simulink model "MCVT_Integration"  (R2020a)
settings: Solver=ode8  StartTime=0.0  StopTime=T_end  FixedStep=dt  RelTol=1e-3  EnableMultiTasking=on
values from neighbouring files (symbols this model uses):
  assumed_power_voltage = 1.25e2    (= 125)    [MCVT_Input_File.m]    (also in MCVT_Run_QuickCheck.m)
  battery_power_toggle = 1    [MCVT_Input_File.m]    (also in MCVT_Run_QuickCheck.m)
  battery_trickle_charge_param = 0.1    [MCVT_Input_File.m]    (also in MCVT_Run_QuickCheck.m)
  battery_trickle_leakage_param = 0.01    [MCVT_Input_File.m]    (also in MCVT_Run_QuickCheck.m)
  damage_case_id = 3    [MCVT_Input_File.m]    (also in MCVT_Run_QuickCheck.m = 1)
  dt = 1/fs    (= 0.001)    [MCVT_Run_QuickCheck.m]    (also in MCVT_Sim_Set.m)
  dt_2 = 1/fs_2    (= 0.001)    [MCVT_Run_QuickCheck.m]    (also in MCVT_Sim_Set.m)
  dt_3 = 1/fs_3    (= 0.001)    [MCVT_Run_QuickCheck.m]    (also in MCVT_Sim_Set.m)
  dt_5 = 1/fs_5    (= 0.005)    [MCVT_Run_QuickCheck.m]    (also in MCVT_Sim_Set.m)
  dt_8 = 1/fs_8    (= 0.05)    [MCVT_Run_QuickCheck.m]    (also in MCVT_Sim_Set.m)
  dt_9 = 1/fs_9    (= 0.001)    [MCVT_Run_QuickCheck.m]    (also in MCVT_Sim_Set.m)
  dust_met_duration = 3    [MCVT_Input_File.m]    (also in MCVT_Run_QuickCheck.m = 2)
  ECLSS_indoor_set_temperature = 298.15    [MCVT_Input_File.m]    (also in MCVT_Run_QuickCheck.m)
  energy_store_max_o = 150    [MCVT_Input_File.m]    (also in MCVT_Run_QuickCheck.m)
  energy_store_o = 120    [MCVT_Input_File.m]    (also in MCVT_Run_QuickCheck.m)
  fs = 1000    [MCVT_Run_QuickCheck.m]    (also in MCVT_Sim_Set.m)
  gain_charge_param = 1    [MCVT_Input_File.m]    (also in MCVT_Run_QuickCheck.m)
  ground_temperature = 373    [MCVT_Habitat_Design_Input_File.m]    (also in MCVT_Run_QuickCheck.m)
  I_max_charge = 800    [MCVT_Input_File.m]    (also in MCVT_Run_QuickCheck.m)
  I_max_discharge = 800    [MCVT_Input_File.m]    (also in MCVT_Run_QuickCheck.m)
  initial_pressure = 1e5    (= 100000)    [MCVT_Input_File.m]    (also in MCVT_Run_QuickCheck.m)
  initial_temperature = 295    [MCVT_Input_File.m]    (also in MCVT_Run_QuickCheck.m)
  interior_leak_rate = 0.00415    [MCVT_Input_File.m]    (also in MCVT_Run_QuickCheck.m)
  launch_dust_accum_start_time = 10    [MCVT_Input_File.m]    (also in MCVT_Run_QuickCheck.m = 2)
  launch_dust_accum_stop_time = 12    [MCVT_Input_File.m]    (also in MCVT_Run_QuickCheck.m = 3)
  meteorite_impact_time = 2    [MCVT_Input_File.m]    (also in MCVT_Run_QuickCheck.m = 1)
  nuclear_power_toggle = 1    [MCVT_Input_File.m]    (also in MCVT_Run_QuickCheck.m)
  print_interval = 30    [MCVT_Input_File.m]
  SOC_lower_thres = 0.5    [MCVT_Input_File.m]    (also in MCVT_Run_QuickCheck.m)
  SOC_upper_thres = 0.9    [MCVT_Input_File.m]    (also in MCVT_Run_QuickCheck.m)
  solar_power_toggle = 1    [MCVT_Input_File.m]    (also in MCVT_Run_QuickCheck.m)
  space_temperature = 4    [MCVT_Habitat_Design_Input_File.m]    (also in MCVT_Run_QuickCheck.m)
  Start_angle = 90    [MCVT_Input_File.m]    (also in MCVT_Run_QuickCheck.m)
undefined symbols (not defined in the file or in its folder): A_flow_restrictor, ACTIVITY_LOOKUP_TABLE, air, Aref, Bmap, Bmap2, Bmap3, comms, DiscIntNeverNeededParam, DmgSPL, dust_parameters, ECLSS_dust_damage_repair_time, ECLSS_heater_capacity, ECLSS_IPS_restrictor, ECLSS_IPS_setpointPressure, ECLSS_paint_damage_repair_time, ECLSS_radiatorLoop_discreteNumber, ECLSS_Vst, emptyActivity, expected_heat_add, FI, habitat, HIEM2SMM, HIEM2STM, HIEMDamageElements, impact_numbering, initial_SPLT_damage_index, input_air, input_Cond, input_CP, input_discretization, input_EV, input_Evap, input_radiatorLoop, K_ECLSS_dust_single, K_ECLSS_paint_single, K_Structure_damage_single, Kref, kW_to_W, meteoroidDustDep, mFRF, ndelays, nominalDustDep, noverlap, nuclear_toggle_gain, output_limits, panel_paint0, Ps0, reference_temperature, RepairTime_ECLSS_factor, Sensor_Location_SMM, SMM_T0, SMM_T1, SMM_T2, SMM_T3, SMM_T4, SMM_T4_0, SMM_T4_1, SMM_T4_2, solar_FDD_K ... (+73)
libraries: neural x350, simulink x39, xpcutilitieslib x7, xpcrtudplib x7, dspstat3 x1
(part 25 of 43)

== system MCVT_Integration/Sys2 - Structural System/Sys2_Structural_System/Fault Detection and Diagnosis (FDD) with Binary Classifer/Pattern Recognition Neural Network/Segment 2 (2)/Layer 2/Delays 1 ==
  "p{2}" : Inport  PortDimensions=20
      mask Display: e = 0.06;c1 = [120 230 180]/255;c2 = ([120 230 180]+0)/(256+128);c3 = ([120 230 180]+128)/(256+128);patch([0 1 1 0],[0 0 1 1],c1);patch([0 1 1 1-e 1-e 0+e],[0 0 1 1-e 0+e 0+e],c2);patch([0 0 1 1-e 0+e 0+e],[0 1 1 1-e 1-e 0+e],c3);plot([0 1 1 0 0],[0 0 1 1 0]);
  mux : Mux  Inputs=1
      mask Display: e = 0.06;c1 = [120 150 230]/255;c2 = ([120 150 230]+0)/383;c3 = ([120 150 230]+128)/383;patch([0 1 1 0],[0 0 1 1],c1);patch([0 1 1 1-e 1-e 0+e],[0 0 1 1-e 0+e 0+e],c2);patch([0 0 1 1-e 0+e 0+e],[0 1 1 1-e 1-e 0+e],c3);plot([0 1 1 0 0],[0 0 1 1 0]);disp('Mux')
  "pd{2,1}" : Outport
      mask Display: e = 0.06;c1 = [120 230 180]/255;c2 = ([120 230 180]+0)/(256+128);c3 = ([120 230 180]+128)/(256+128);patch([0 1 1 0],[0 0 1 1],c1);patch([0 1 1 1-e 1-e 0+e],[0 0 1 1-e 0+e 0+e],c2);patch([0 0 1 1-e 0+e 0+e],[0 1 1 1-e 1-e 0+e],c3);plot([0 1 1 0 0],[0 0 1 1 0]);
  wires:
  "p{2}"/1 -> mux/1
  mux/1 -> "pd{2,1}"/1

== system MCVT_Integration/Sys2 - Structural System/Sys2_Structural_System/Fault Detection and Diagnosis (FDD) with Binary Classifer/Pattern Recognition Neural Network/Segment 2 (2)/Layer 2/LW{2,1} ==
  "ad{2,1}" : Inport  PortDimensions=20
      mask Display: e = 0.06;c1 = [120 230 180]/255;c2 = ([120 230 180]+0)/(256+128);c3 = ([120 230 180]+128)/(256+128);patch([0 1 1 0],[0 0 1 1],c1);patch([0 1 1 1-e 1-e 0+e],[0 0 1 1-e 0+e 0+e],c2);patch([0 0 1 1-e 0+e 0+e],[0 1 1 1-e 1-e 0+e],c3);plot([0 1 1 0 0],[0 0 1 1 0]);
  "IW{2,1}(1,:)'" : Constant  Value=[-0.0131519501662464843860789187601767480373382568359375;-0.2283490556409899785439421293631312437355518341064453125;-0.289726021936160205161314706856501288712024688720703125;-0.610136834219936030621056488598696887493133544921875;-0.2034645657655405892594302486031665466725826263427734375;-0.1781002138410512636301774591629509814083576202392578125;-0.29252056118321412281346738382126204669475555419921875;0.62775860490154566928566737260553054511547088623046875;0.436139315774980607098854079595184884965419769287109375;-0.09668622512830470083766698508043191395699977874755859375;-0.117092883490729604556435106132994405925273895263671875;-0.50352244145249358542315576414694078266620635986328125;0.447763606238912259538409443848649971187114715576171875;0.16290226867061274873549336916767060756683349609375;-0.07475694900080988303248119564159424044191837310791015625;0.10059141232051337089270504066007561050355434417724609375;0.392832502066031874488061248484882526099681854248046875;-0.37563150969789649291641353556769900023937225341796875;0.6032049190998052257128847486455924808979034423828125;0.5332559068353577291787814829149283468723297119140625]
      mask Display: e = 0.06;c1 = [120 150 230]/255;c2 = ([120 150 230]+0)/383;c3 = ([120 150 230]+128)/383;patch([0 1 1 0],[0 0 1 1],c1);patch([0 1 1 1-e 1-e 0+e],[0 0 1 1-e 0+e 0+e],c2);patch([0 0 1 1-e 0+e 0+e],[0 1 1 1-e 1-e 0+e],c3);plot([0 1 1 0 0],[0 0 1 1 0]);disp('weights');
  "IW{2,1}(10,:)'" : Constant  Value=[0.197300923241983838796187455955077894032001495361328125;-0.09049196986361050798475247347596450708806514739990234375;-0.14234633867032464582536022135172970592975616455078125;-0.3499922447867491737127920714556239545345306396484375;-0.12360098816561958245952013157875626347959041595458984375;-0.53617351984011907273242059090989641845226287841796875;-0.63019586873835731211102029192261397838592529296875;-0.59953072347420388599203988633234985172748565673828125;-0.0963323722927145464911546923758578486740589141845703125;0.31476140520503637088012283129501156508922576904296875;0.1658873255039448324499318232483346946537494659423828125;-0.5239396673414631333542956781457178294658660888671875;-0.359447552274538340011389436767785809934139251708984375;-0.52141836566016053211569669656455516815185546875;0.1987488184022156989527729820110835134983062744140625;0.13545688063019600821945687130209989845752716064453125;-0.390371505202162349945638197823427617549896240234375;-0.330714938560074944717825928819365799427032470703125;0.195111837513341768168828593843500129878520965576171875;0.477428590936654184684329038645955733954906463623046875]
      mask Display: e = 0.06;c1 = [120 150 230]/255;c2 = ([120 150 230]+0)/383;c3 = ([120 150 230]+128)/383;patch([0 1 1 0],[0 0 1 1],c1);patch([0 1 1 1-e 1-e 0+e],[0 0 1 1-e 0+e 0+e],c2);patch([0 0 1 1-e 0+e 0+e],[0 1 1 1-e 1-e 0+e],c3);plot([0 1 1 0 0],[0 0 1 1 0]);disp('weights');
  "IW{2,1}(11,:)'" : Constant  Value=[-0.43816409613136875211836240850971080362796783447265625;0.050414382838295558608709967529648565687239170074462890625;0.3709541683366754938333542668260633945465087890625;0.362179073798906348002901722793467342853546142578125;-0.477214101448056371435058053975808434188365936279296875;0.09154699814056588280664072954095900058746337890625;-0.507130106053504281504729078733362257480621337890625;-0.1507137484925420156667286164520191960036754608154296875;0.275939301654270441321870066531118936836719512939453125;-0.2303026309926386738791137531734420917928218841552734375;-0.370681237269094354669363156062900088727474212646484375;0.26264946901190366901346351369284093379974365234375;-0.35779494535728095794269165708101354539394378662109375;0.276947799384692883162273346897563897073268890380859375;-0.529013627469939518022101765382103621959686279296875;0.498452822401328765611339122187928296625614166259765625;-0.52921250233643568616770380685920827090740203857421875;-0.2138755050931575862893652129059773869812488555908203125;0.1237822832115683391318583517204388044774532318115234375;-0.52223863473583331806793239593389444053173065185546875]
      mask Display: e = 0.06;c1 = [120 150 230]/255;c2 = ([120 150 230]+0)/383;c3 = ([120 150 230]+128)/383;patch([0 1 1 0],[0 0 1 1],c1);patch([0 1 1 1-e 1-e 0+e],[0 0 1 1-e 0+e 0+e],c2);patch([0 0 1 1-e 0+e 0+e],[0 1 1 1-e 1-e 0+e],c3);plot([0 1 1 0 0],[0 0 1 1 0]);disp('weights');
  "IW{2,1}(12,:)'" : Constant  Value=[-0.154179751547239674014377897037775255739688873291015625;0.24132279555188151931588436127640306949615478515625;-0.1907832451890008884642924158470123074948787689208984375;-0.1898471638859701438040161747267120517790317535400390625;0.08850062513875656122763757593929767608642578125;-0.09830670129288728020799936757612158544361591339111328125;-0.3458340449024610574468852064455859363079071044921875;0.58622057419220741092402704452979378402233123779296875;-0.096689363847558051912045584685984067618846893310546875;-0.121243727115140698114004180752090178430080413818359375;-0.34262826548283153993423866268130950629711151123046875;0.42695656487580613269727791703189723193645477294921875;0.0409180102003559864609627538811764679849147796630859375;-0.215755452435445416181636346664163284003734588623046875;-0.368713595036108288116594167149742133915424346923828125;-0.63218708471201845444653599770390428602695465087890625;0.54504381690632286439068820982356555759906768798828125;0.5238956902929248204969780999817885458469390869140625;0.58609328606454325072405708851874805986881256103515625;0.43090807344199133499529352775425650179386138916015625]
      mask Display: e = 0.06;c1 = [120 150 230]/255;c2 = ([120 150 230]+0)/383;c3 = ([120 150 230]+128)/383;patch([0 1 1 0],[0 0 1 1],c1);patch([0 1 1 1-e 1-e 0+e],[0 0 1 1-e 0+e 0+e],c2);patch([0 0 1 1-e 0+e 0+e],[0 1 1 1-e 1-e 0+e],c3);plot([0 1 1 0 0],[0 0 1 1 0]);disp('weights');
  "IW{2,1}(13,:)'" : Constant  Value=[-0.30546889022732603979903842628118582069873809814453125;0.51596770552269377585474785519181750714778900146484375;-0.34669813473577004714343274827115237712860107421875;0.12420386955850241939014466652224655263125896453857421875;0.63521116212299399972351920951041392982006072998046875;0.141702815706782114801853822427801787853240966796875;-0.04114993903501988625759366868805955164134502410888671875;-0.01341912357002485005341707591242084163241088390350341796875;-0.2256961036674860476836101952358148992061614990234375;0.07905006322392633955065122108862851746380329132080078125;0.789135755776883218715056500514037907123565673828125;0.00699798297809421480930591741298485430888831615447998046875;0.7010967977420377028607845204533077776432037353515625;0.1653077210484481252006361273743095807731151580810546875;-0.138169087998334438882608310450450517237186431884765625;0.32630544085148105448723754307138733565807342529296875;-0.041991616347027027611460425760014913976192474365234375;0.635256180584609086992031734553165733814239501953125;-0.1329167055636059913137358989843050949275493621826171875;0.031908811545528194264509380673189298249781131744384765625]
      mask Display: e = 0.06;c1 = [120 150 230]/255;c2 = ([120 150 230]+0)/383;c3 = ([120 150 230]+128)/383;patch([0 1 1 0],[0 0 1 1],c1);patch([0 1 1 1-e 1-e 0+e],[0 0 1 1-e 0+e 0+e],c2);patch([0 0 1 1-e 0+e 0+e],[0 1 1 1-e 1-e 0+e],c3);plot([0 1 1 0 0],[0 0 1 1 0]);disp('weights');
  "IW{2,1}(14,:)'" : Constant  Value=[0.67168627283706328068291213639895431697368621826171875;0.1960581153137800625163578160936594940721988677978515625;0.1827421028051608165032604347288724966347217559814453125;-0.197999380927649848427307688325527124106884002685546875;-0.5284048661146687475564931446569971740245819091796875;-0.52095844209163544302754189629922620952129364013671875;0.2375769278955271179842867468323674984276294708251953125;0.413627245104900620464860594438505358994007110595703125;0.1650150353793438939842275203773169778287410736083984375;0.00104023284521608254567237583643191101145930588245391845703125;0.4547232127345341989865801224368624389171600341796875;0.1755674189985755584419990782407694496214389801025390625;0.396113807751520552447033196585834957659244537353515625;-0.37449472969020780599436193369911052286624908447265625;0.548398537903627580902821136987768113613128662109375;0.3822967344072323303549865158856846392154693603515625;0.258528703254273295453202763383160345256328582763671875;0.14519592981383999674704909921274520456790924072265625;0.333280739538195813764787089894525706768035888671875;-0.418880074668204371857171963711152784526348114013671875]
      mask Display: e = 0.06;c1 = [120 150 230]/255;c2 = ([120 150 230]+0)/383;c3 = ([120 150 230]+128)/383;patch([0 1 1 0],[0 0 1 1],c1);patch([0 1 1 1-e 1-e 0+e],[0 0 1 1-e 0+e 0+e],c2);patch([0 0 1 1-e 0+e 0+e],[0 1 1 1-e 1-e 0+e],c3);plot([0 1 1 0 0],[0 0 1 1 0]);disp('weights');
  "IW{2,1}(15,:)'" : Constant  Value=[0.204965259719101233759630531494622118771076202392578125;-0.457528895464862361830427062159287743270397186279296875;0.396842705297933140418109587699291296303272247314453125;-0.209056454484637221913345683788065798580646514892578125;0.39452448007547558095353679163963533937931060791015625;-0.00080403082643378041664827815537819333258084952831268310546875;-0.30421576480985346169205740807228721678256988525390625;-0.40593922296600981614034253652789629995822906494140625;0.167199455682886022334088238494587130844593048095703125;0.37516990390103444052982695211539976298809051513671875;0.04725408586966405033447102823629393242299556732177734375;-0.2294834142945360311305336153964162804186344146728515625;0.055303747495165346548251505964799434877932071685791015625;-0.58871003988118875138724206408369354903697967529296875;0.63547097898882476396664742424036376178264617919921875;-0.5822402191211561994776957362773828208446502685546875;0.386993895216280192617119837450445629656314849853515625;0.322000985675978956823684029586729593575000762939453125;-0.44334798489222648765206713505904190242290496826171875;-0.289612766679017308302235278461012057960033416748046875]
      mask Display: e = 0.06;c1 = [120 150 230]/255;c2 = ([120 150 230]+0)/383;c3 = ([120 150 230]+128)/383;patch([0 1 1 0],[0 0 1 1],c1);patch([0 1 1 1-e 1-e 0+e],[0 0 1 1-e 0+e 0+e],c2);patch([0 0 1 1-e 0+e 0+e],[0 1 1 1-e 1-e 0+e],c3);plot([0 1 1 0 0],[0 0 1 1 0]);disp('weights');
  "IW{2,1}(16,:)'" : Constant  Value=[0.5086877522888617608742833908763714134693145751953125;0.155454499671700385743378092229249887168407440185546875;0.298448023683270335926209781973739154636859893798828125;0.21427800561056697947748261867673136293888092041015625;0.255418943293809486139167574947350658476352691650390625;0.2022610655742179408544956231708056293427944183349609375;0.566119820861264333444751173374243080615997314453125;0.52729264426775224183785439890925772488117218017578125;0.013144346486769355186741137231365428306162357330322265625;-0.40351641591171005796212511995690874755382537841796875;0.329441369614369872209636014304123818874359130859375;0.292127223318678741481591032425058074295520782470703125;0.409605478422182367470583130852901376783847808837890625;-0.480182871235225350314834713572054170072078704833984375;-0.5012470349158772986442045294097624719142913818359375;0.1676099786342380715264965829192078672349452972412109375;0.1959335407295708930686117810182622633874416351318359375;-0.447260994875497031042499429531744681298732757568359375;0.51517899022007984655147083685733377933502197265625;-0.340207096308376477633572676495532505214214324951171875]
      mask Display: e = 0.06;c1 = [120 150 230]/255;c2 = ([120 150 230]+0)/383;c3 = ([120 150 230]+128)/383;patch([0 1 1 0],[0 0 1 1],c1);patch([0 1 1 1-e 1-e 0+e],[0 0 1 1-e 0+e 0+e],c2);patch([0 0 1 1-e 0+e 0+e],[0 1 1 1-e 1-e 0+e],c3);plot([0 1 1 0 0],[0 0 1 1 0]);disp('weights');
  "IW{2,1}(17,:)'" : Constant  Value=[0.2441649229961604106176054074239800684154033660888671875;-0.44964204744072777675256702423212118446826934814453125;-0.475088415645347483806659738547750748693943023681640625;-0.195526594771391282190364790949388407170772552490234375;0.29813918813556294917788136444869451224803924560546875;-0.4409374283631042334974381446954794228076934814453125;0.5065848325108703864572134989430196583271026611328125;0.35097759710480713746250103213242255151271820068359375;-0.2567248005003490707309765639365650713443756103515625;0.38262506906693538599739667915855534374713897705078125;0.1345017254853722865615139880901551805436611175537109375;0.51895290240209501764212518537533469498157501220703125;0.4519398832964345302798392367549240589141845703125;0.133895624946028413138066071041976101696491241455078125;-0.2060589941360758403643416158956824801862239837646484375;-0.397105489244936660231388714237255044281482696533203125;-0.4186089314300984742800437743426300585269927978515625;0.426739301003949578205975967648555524647235870361328125;0.446382722894400008062376628004130907356739044189453125;-0.213927716619095209527046108632930554449558258056640625]
      mask Display: e = 0.06;c1 = [120 150 230]/255;c2 = ([120 150 230]+0)/383;c3 = ([120 150 230]+128)/383;patch([0 1 1 0],[0 0 1 1],c1);patch([0 1 1 1-e 1-e 0+e],[0 0 1 1-e 0+e 0+e],c2);patch([0 0 1 1-e 0+e 0+e],[0 1 1 1-e 1-e 0+e],c3);plot([0 1 1 0 0],[0 0 1 1 0]);disp('weights');
  "IW{2,1}(18,:)'" : Constant  Value=[-0.0272818523825677609895290487429519998840987682342529296875;-0.1992454448341149098045121945688151754438877105712890625;0.00399563298715272137429099785776998032815754413604736328125;-0.49122836298554217737688531997264362871646881103515625;0.26900137384623068470546058961190283298492431640625;0.419204027612011753678444847537321038544178009033203125;-0.56941485889710496959281726958579383790493011474609375;0.59417278501397008394491194849251769483089447021484375;-0.01317092597891093841422360810611280612647533416748046875;0.498478065508463463739730059387511573731899261474609375;-0.1365275002181844110626940391739481128752231597900390625;0.349094615244758987859796661723521538078784942626953125;0.333932621740755941619482882742886431515216827392578125;0.236908066657262217358237421649391762912273406982421875;-0.272717938937628023854387038227287121117115020751953125;-0.267866598781879738933042744974954985082149505615234375;0.47520848350665001458281722079846076667308807373046875;-0.1413815765253161049752606004403787665069103240966796875;-0.28032347811350588617784751477302052080631256103515625;-0.6554303965414323140947772117215208709239959716796875]
      mask Display: e = 0.06;c1 = [120 150 230]/255;c2 = ([120 150 230]+0)/383;c3 = ([120 150 230]+128)/383;patch([0 1 1 0],[0 0 1 1],c1);patch([0 1 1 1-e 1-e 0+e],[0 0 1 1-e 0+e 0+e],c2);patch([0 0 1 1-e 0+e 0+e],[0 1 1 1-e 1-e 0+e],c3);plot([0 1 1 0 0],[0 0 1 1 0]);disp('weights');
  "IW{2,1}(19,:)'" : Constant  Value=[-0.412984680238038748267825894799898378551006317138671875;-0.01197734433299585919685537049872436909936368465423583984375;-0.41675158842994586283481339705758728086948394775390625;0.572292832534660345089605471002869307994842529296875;-0.59742353013398930006161435812828131020069122314453125;0.320138578299695664153290408648899756371974945068359375;-0.23180110089244287951970591166173107922077178955078125;-0.0032164649152252818876951412363496274338103830814361572265625;-0.04897654664051438733185506180234369821846485137939453125;0.27541941282966686532773792350781150162220001220703125;-0.185825929747873541675318165289354510605335235595703125;0.096966390649146472213715242105536162853240966796875;0.54300070707530168068188913821359165012836456298828125;0.6121233500942977467929040358285419642925262451171875;0.53229438575759047136415347267757169902324676513671875;0.1371221791612284024441947849481948651373386383056640625;0.337732805587329820706798955143312923610210418701171875;-0.471249189997828554776759801825392059981822967529296875;0.1414943335377516209216963716244208626449108123779296875;-0.260606610726190235372001779978745616972446441650390625]
      mask Display: e = 0.06;c1 = [120 150 230]/255;c2 = ([120 150 230]+0)/383;c3 = ([120 150 230]+128)/383;patch([0 1 1 0],[0 0 1 1],c1);patch([0 1 1 1-e 1-e 0+e],[0 0 1 1-e 0+e 0+e],c2);patch([0 0 1 1-e 0+e 0+e],[0 1 1 1-e 1-e 0+e],c3);plot([0 1 1 0 0],[0 0 1 1 0]);disp('weights');
  "IW{2,1}(2,:)'" : Constant  Value=[0.333457618335939509623955245842807926237583160400390625;0.31260328922987612454420514040975831449031829833984375;0.079716604354632514262135600802139379084110260009765625;0.26287435946747350623553529658238403499126434326171875;0.307987751629796802088634422034374438226222991943359375;-0.434530480747118363904490934146451763808727264404296875;-0.1955650245888486959966456879556062631309032440185546875;0.0316630319989695541149643531753099523484706878662109375;0.149008003242111553010573743449640460312366485595703125;-0.1762212999549400971321944098235690034925937652587890625;-0.549049382692389453808345933794043958187103271484375;-0.007927998603949625022746516833649366162717342376708984375;0.04347451355695024222125510959813254885375499725341796875;0.5942988052607638405078205323661677539348602294921875;0.4983561964854132941837860926170833408832550048828125;-0.1307877313259060148009638169241952709853649139404296875;0.58851957543416444362804895717999897897243499755859375;0.58966588037519584997170341011951677501201629638671875;0.27866051602814623233683732905774377286434173583984375;0.5400702859147890055879770443425513803958892822265625]
      mask Display: e = 0.06;c1 = [120 150 230]/255;c2 = ([120 150 230]+0)/383;c3 = ([120 150 230]+128)/383;patch([0 1 1 0],[0 0 1 1],c1);patch([0 1 1 1-e 1-e 0+e],[0 0 1 1-e 0+e 0+e],c2);patch([0 0 1 1-e 0+e 0+e],[0 1 1 1-e 1-e 0+e],c3);plot([0 1 1 0 0],[0 0 1 1 0]);disp('weights');
  "IW{2,1}(20,:)'" : Constant  Value=[0.37774749543291308295778208048432134091854095458984375;0.450209832735798787251013663990306667983531951904296875;-0.01815944383427547936804558048606850206851959228515625;-0.322358661882434838208411065352265723049640655517578125;0.3101929148373734346222363456035964190959930419921875;0.28410063058146717285268323394120670855045318603515625;-0.261028404079189424447093870185199193656444549560546875;0.0238287955920568998713360286956230993382632732391357421875;0.1632484893172323758836483875711564905941486358642578125;-0.59584478130304330267819068467360921204090118408203125;-0.51739031850990857552829993437626399099826812744140625;0.45758039328849431370827005594037473201751708984375;0.497997300177656310182783272466622292995452880859375;0.34127307260028449942268480299389921128749847412109375;-0.39211144835421907206551850322284735739231109619140625;0.187366874416645179390883413361734710633754730224609375;-0.527069786600139256194097470142878592014312744140625;0.066239801981011814380195801277295686304569244384765625;0.49808832985547246696711454205797053873538970947265625;0.171781567452022498887487245156080462038516998291015625]
      mask Display: e = 0.06;c1 = [120 150 230]/255;c2 = ([120 150 230]+0)/383;c3 = ([120 150 230]+128)/383;patch([0 1 1 0],[0 0 1 1],c1);patch([0 1 1 1-e 1-e 0+e],[0 0 1 1-e 0+e 0+e],c2);patch([0 0 1 1-e 0+e 0+e],[0 1 1 1-e 1-e 0+e],c3);plot([0 1 1 0 0],[0 0 1 1 0]);disp('weights');
  "IW{2,1}(3,:)'" : Constant  Value=[0.35439732233827825336192063332418911159038543701171875;-0.313091430040572660242759184257010929286479949951171875;0.12452339499336524675232595882334862835705280303955078125;0.40565264515026833169741848905687220394611358642578125;0.57658539475180392575026644408353604376316070556640625;-0.08658277145044911138338505907086073420941829681396484375;-0.596227988176643908246887804125435650348663330078125;0.268082521162564424432872556280926801264286041259765625;-0.07489967915217420058038300112457363866269588470458984375;0.2481297207565101581305810896083130501210689544677734375;0.2074183065167887474178343154562753625214099884033203125;0.294677077928551744978591386825428344309329986572265625;-0.56237737470870763356600718907429836690425872802734375;-0.183562744032721936182639410617412067949771881103515625;0.481266272701765307484578215735382400453090667724609375;-0.33702111782774923920413812084007076919078826904296875;-0.487107004605000282193572047617635689675807952880859375;0.4731483956925763845191568179870955646038055419921875;-0.036816182760127623418977549363262369297444820404052734375;-0.34504886566515347023909043855383060872554779052734375]
      mask Display: e = 0.06;c1 = [120 150 230]/255;c2 = ([120 150 230]+0)/383;c3 = ([120 150 230]+128)/383;patch([0 1 1 0],[0 0 1 1],c1);patch([0 1 1 1-e 1-e 0+e],[0 0 1 1-e 0+e 0+e],c2);patch([0 0 1 1-e 0+e 0+e],[0 1 1 1-e 1-e 0+e],c3);plot([0 1 1 0 0],[0 0 1 1 0]);disp('weights');
  "IW{2,1}(4,:)'" : Constant  Value=[-0.386695633926689819048760909936390817165374755859375;0.255337156497296657864382041225326247513294219970703125;0.311559038575999147635542385614826343953609466552734375;-0.171833764302430302972624076573993079364299774169921875;0.42815980888437732421181181052816100418567657470703125;0.1841038622537757574182393227602005936205387115478515625;-0.514405240272733710327202061307616531848907470703125;-0.6114171015452611879226196833769790828227996826171875;0.2549273615071883103411209958721883594989776611328125;-0.358671088136504001564475174745894037187099456787109375;0.2271734734148021794819527485742582939565181732177734375;-0.50116453450551678994173698811209760606288909912109375;0.0279531759745337386735553053540570545010268688201904296875;0.2470897484313333281047420086906640790402889251708984375;-0.161814456109800886007832332325051538646221160888671875;-0.53806900757107822830249688195181079208850860595703125;-0.427958005212859593502372490547713823616504669189453125;0.388009745019373741836687941031414084136486053466796875;0.477700509700453801453789992592646740376949310302734375;-0.102658369668155058374026111778221093118190765380859375]
      mask Display: e = 0.06;c1 = [120 150 230]/255;c2 = ([120 150 230]+0)/383;c3 = ([120 150 230]+128)/383;patch([0 1 1 0],[0 0 1 1],c1);patch([0 1 1 1-e 1-e 0+e],[0 0 1 1-e 0+e 0+e],c2);patch([0 0 1 1-e 0+e 0+e],[0 1 1 1-e 1-e 0+e],c3);plot([0 1 1 0 0],[0 0 1 1 0]);disp('weights');
  "IW{2,1}(5,:)'" : Constant  Value=[-0.2183548213776102253635968963862978853285312652587890625;-0.06686080163682102395217299317664583213627338409423828125;-0.3059856159244294104837535996921360492706298828125;0.50628347562946640625369809640687890350818634033203125;-0.059884992527261891870349330702083534561097621917724609375;-0.42947409214421894407820445849210955202579498291015625;0.00577882712320255086113629516830769716762006282806396484375;0.57894313286325782552665941693703643977642059326171875;-0.228878604361737847394664413513964973390102386474609375;0.38508070925969029030255796897108666598796844482421875;-0.56889230433675719300623541130335070192813873291015625;-0.0214034368517706762025110123204285628162324428558349609375;0.2011680864736654361646372990435338579118251800537109375;0.07659518359337459669777814497138024307787418365478515625;0.58426152416850152260252571068122051656246185302734375;0.261441142296763684438332120407721959054470062255859375;-0.473890859988484181020140795226325280964374542236328125;-0.038417399100486700203038736844973755069077014923095703125;0.59236811667310129703167831394239328801631927490234375;-0.397314077343586535828734440656262449920177459716796875]
      mask Display: e = 0.06;c1 = [120 150 230]/255;c2 = ([120 150 230]+0)/383;c3 = ([120 150 230]+128)/383;patch([0 1 1 0],[0 0 1 1],c1);patch([0 1 1 1-e 1-e 0+e],[0 0 1 1-e 0+e 0+e],c2);patch([0 0 1 1-e 0+e 0+e],[0 1 1 1-e 1-e 0+e],c3);plot([0 1 1 0 0],[0 0 1 1 0]);disp('weights');
  "IW{2,1}(6,:)'" : Constant  Value=[-0.52038816182933345100281030681799165904521942138671875;-0.47827678462462552833045492661767639219760894775390625;-0.012618800528797657900526019147946499288082122802734375;-0.51684940007109048121236583028803579509258270263671875;0.5007574950134270341806086435099132359027862548828125;0.44522443493419039395320169205660931766033172607421875;-0.03644083145788785949026333810252253897488117218017578125;0.51043943242322165065871786282514221966266632080078125;-0.378811203678414809559171771979890763759613037109375;0.5236463638309718504615375422872602939605712890625;0.0476719440912919123487512251813313923776149749755859375;-0.11901798838266962332621545783695182763040065765380859375;-0.368746150437196662519312440053909085690975189208984375;-0.149549170352473448364349906114512123167514801025390625;-0.08908356480650823916445091299465275369584560394287109375;-0.337019281818837501685237612036871723830699920654296875;-0.00727271919906051500515076213559950701892375946044921875;-0.58588010866313255942117166341631673276424407958984375;-0.039562114831076723719949228552650311030447483062744140625;0.327220002377005492188999369318480603396892547607421875]
      mask Display: e = 0.06;c1 = [120 150 230]/255;c2 = ([120 150 230]+0)/383;c3 = ([120 150 230]+128)/383;patch([0 1 1 0],[0 0 1 1],c1);patch([0 1 1 1-e 1-e 0+e],[0 0 1 1-e 0+e 0+e],c2);patch([0 0 1 1-e 0+e 0+e],[0 1 1 1-e 1-e 0+e],c3);plot([0 1 1 0 0],[0 0 1 1 0]);disp('weights');
  "IW{2,1}(7,:)'" : Constant  Value=[-0.427274467634338350752187807302107103168964385986328125;0.349354643114616225663127124789752997457981109619140625;-0.45156490884748212710775305822608061134815216064453125;-0.247476066573397657233357449513277970254421234130859375;-0.333650397681086896550795017901691608130931854248046875;-0.50567896573378090518957606036565266549587249755859375;0.2867711824106915496912506569060496985912322998046875;0.084802900833068350916477129430859349668025970458984375;-0.477034292082058553052803517857682891190052032470703125;0.10820511835584674387167325448899646289646625518798828125;0.470801628314395426144756129360757768154144287109375;0.26092044910748846131554046223754994571208953857421875;7.601676874353815831220071430607276852242648601531982421875e-05;-0.1250307582448890730830726170097477734088897705078125;0.4440635884883621375962547972449101507663726806640625;-0.310202392049235997628642280687927268445491790771484375;-0.1241160455325439337936899164560600183904170989990234375;0.52673883361213824638724645410547964274883270263671875;0.4924780110124926668646594407618977129459381103515625;-0.493337358611206588943076667419518344104290008544921875]
      mask Display: e = 0.06;c1 = [120 150 230]/255;c2 = ([120 150 230]+0)/383;c3 = ([120 150 230]+128)/383;patch([0 1 1 0],[0 0 1 1],c1);patch([0 1 1 1-e 1-e 0+e],[0 0 1 1-e 0+e 0+e],c2);patch([0 0 1 1-e 0+e 0+e],[0 1 1 1-e 1-e 0+e],c3);plot([0 1 1 0 0],[0 0 1 1 0]);disp('weights');
  "IW{2,1}(8,:)'" : Constant  Value=[0.2206718899939234812546118291720631532371044158935546875;-0.491786404436899526881887823037686757743358612060546875;-0.15081035662256370155631657326011918485164642333984375;0.269003332273807183039338042362942360341548919677734375;-0.267659082437678164101413358366698957979679107666015625;-0.3705729579415730778890747387777082622051239013671875;-0.0301870488982296895918988610674205119721591472625732421875;-0.07900287876336682668654276540110004134476184844970703125;0.5322020841328576334916533596697263419628143310546875;0.348935966720793355744234531812253408133983612060546875;0.53035094339864752299718020367436110973358154296875;0.055806070148112042750287997705527232028543949127197265625;0.115159355750261427342451270305900834500789642333984375;-0.324039560187445896399793809905531816184520721435546875;0.5739671701906299272621936324867419898509979248046875;0.09818602109514940057266585426987148821353912353515625;0.500286843329077246522729183197952806949615478515625;-0.1308499825780572722866423873711028136312961578369140625;-0.57865975211681963674692497079377062618732452392578125;-0.55114518217831420798802355420775711536407470703125]
      mask Display: e = 0.06;c1 = [120 150 230]/255;c2 = ([120 150 230]+0)/383;c3 = ([120 150 230]+128)/383;patch([0 1 1 0],[0 0 1 1],c1);patch([0 1 1 1-e 1-e 0+e],[0 0 1 1-e 0+e 0+e],c2);patch([0 0 1 1-e 0+e 0+e],[0 1 1 1-e 1-e 0+e],c3);plot([0 1 1 0 0],[0 0 1 1 0]);disp('weights');
  "IW{2,1}(9,:)'" : Constant  Value=[0.3285680987959052057334474739036522805690765380859375;-0.625155647366722799773697261116467416286468505859375;0.497450645624185394666483261971734464168548583984375;-0.11731213776090250455741426094391499646008014678955078125;0.3868157822455235983483134987181983888149261474609375;-0.30982625859112911115289534791372716426849365234375;-0.024494204292524524024798893151455558836460113525390625;-0.1524419320254502829836695809717639349400997161865234375;0.2254339981644810919636512380748172290623188018798828125;-0.12036533911250353601207763176716980524361133575439453125;-0.41302902428931476119799981461255811154842376708984375;0.55808267212438755766612530351267196238040924072265625;-0.352172131086202544469898612078395672142505645751953125;-0.60351489861987861473835437209345400333404541015625;-0.091047490011318010783014642584021203219890594482421875;-0.71867826281836577617667671802337281405925750732421875;0.1197369076726642334040917603488196618854999542236328125;-0.06723370284726092049876200462676933966577053070068359375;0.028071032978776909450058241191072738729417324066162109375;0.185028771230855204610321607106016017496585845947265625]
      mask Display: e = 0.06;c1 = [120 150 230]/255;c2 = ([120 150 230]+0)/383;c3 = ([120 150 230]+128)/383;patch([0 1 1 0],[0 0 1 1],c1);patch([0 1 1 1-e 1-e 0+e],[0 0 1 1-e 0+e 0+e],c2);patch([0 0 1 1-e 0+e 0+e],[0 1 1 1-e 1-e 0+e],c3);plot([0 1 1 0 0],[0 0 1 1 0]);disp('weights');
  Mux : Mux  Inputs=20
      mask Display: e = 0.06;c1 = [120 150 230]/255;c2 = ([120 150 230]+0)/383;c3 = ([120 150 230]+128)/383;patch([0 1 1 0],[0 0 1 1],c1);patch([0 1 1 1-e 1-e 0+e],[0 0 1 1-e 0+e 0+e],c2);patch([0 0 1 1-e 0+e 0+e],[0 1 1 1-e 1-e 0+e],c3);plot([0 1 1 0 0],[0 0 1 1 0]);disp('Mux');
  dotprod{1..20} (20 alike) : neural/Weight Functions/dotprod
  "lz{2,1}" : Outport
      mask Display: e = 0.06;c1 = [120 230 180]/255;c2 = ([120 230 180]+0)/(256+128);c3 = ([120 230 180]+128)/(256+128);patch([0 1 1 0],[0 0 1 1],c1);patch([0 1 1 1-e 1-e 0+e],[0 0 1 1-e 0+e 0+e],c2);patch([0 0 1 1-e 0+e 0+e],[0 1 1 1-e 1-e 0+e],c3);plot([0 1 1 0 0],[0 0 1 1 0]);
  wires:
  "IW{2,1}(1,:)'"/1 -> dotprod1/1
  "ad{2,1}"/1 -> dotprod1/2, dotprod2/2, dotprod3/2, dotprod4/2, dotprod5/2, dotprod6/2, dotprod7/2, dotprod8/2, dotprod9/2, dotprod10/2, dotprod11/2, dotprod12/2, dotprod13/2, dotprod14/2, dotprod15/2, dotprod16/2, dotprod17/2, dotprod18/2, dotprod19/2, dotprod20/2
  dotprod1/1 -> Mux/1
  "IW{2,1}(2,:)'"/1 -> dotprod2/1
  dotprod2/1 -> Mux/2
  "IW{2,1}(3,:)'"/1 -> dotprod3/1
  dotprod3/1 -> Mux/3
  "IW{2,1}(4,:)'"/1 -> dotprod4/1
  dotprod4/1 -> Mux/4
  "IW{2,1}(5,:)'"/1 -> dotprod5/1
  dotprod5/1 -> Mux/5
  "IW{2,1}(6,:)'"/1 -> dotprod6/1
  dotprod6/1 -> Mux/6
  "IW{2,1}(7,:)'"/1 -> dotprod7/1
  dotprod7/1 -> Mux/7
  "IW{2,1}(8,:)'"/1 -> dotprod8/1
  dotprod8/1 -> Mux/8
  "IW{2,1}(9,:)'"/1 -> dotprod9/1
  dotprod9/1 -> Mux/9
  "IW{2,1}(10,:)'"/1 -> dotprod10/1
  dotprod10/1 -> Mux/10
  "IW{2,1}(11,:)'"/1 -> dotprod11/1
  dotprod11/1 -> Mux/11
  "IW{2,1}(12,:)'"/1 -> dotprod12/1
  dotprod12/1 -> Mux/12
  "IW{2,1}(13,:)'"/1 -> dotprod13/1
  dotprod13/1 -> Mux/13
  "IW{2,1}(14,:)'"/1 -> dotprod14/1
  dotprod14/1 -> Mux/14
  "IW{2,1}(15,:)'"/1 -> dotprod15/1
  dotprod15/1 -> Mux/15
  "IW{2,1}(16,:)'"/1 -> dotprod16/1
  dotprod16/1 -> Mux/16
  "IW{2,1}(17,:)'"/1 -> dotprod17/1
  dotprod17/1 -> Mux/17
  "IW{2,1}(18,:)'"/1 -> dotprod18/1
  dotprod18/1 -> Mux/18
  "IW{2,1}(19,:)'"/1 -> dotprod19/1
  dotprod19/1 -> Mux/19
  "IW{2,1}(20,:)'"/1 -> dotprod20/1
  dotprod20/1 -> Mux/20
  Mux/1 -> "lz{2,1}"/1

== system MCVT_Integration/Sys2 - Structural System/Sys2_Structural_System/Fault Detection and Diagnosis (FDD) with Binary Classifer/Pattern Recognition Neural Network/Segment 2 (2)/Layer 3/Delays 1 ==
  "p{3}" : Inport  PortDimensions=20
      mask Display: e = 0.06;c1 = [120 230 180]/255;c2 = ([120 230 180]+0)/(256+128);c3 = ([120 230 180]+128)/(256+128);patch([0 1 1 0],[0 0 1 1],c1);patch([0 1 1 1-e 1-e 0+e],[0 0 1 1-e 0+e 0+e],c2);patch([0 0 1 1-e 0+e 0+e],[0 1 1 1-e 1-e 0+e],c3);plot([0 1 1 0 0],[0 0 1 1 0]);
  mux : Mux  Inputs=1
      mask Display: e = 0.06;c1 = [120 150 230]/255;c2 = ([120 150 230]+0)/383;c3 = ([120 150 230]+128)/383;patch([0 1 1 0],[0 0 1 1],c1);patch([0 1 1 1-e 1-e 0+e],[0 0 1 1-e 0+e 0+e],c2);patch([0 0 1 1-e 0+e 0+e],[0 1 1 1-e 1-e 0+e],c3);plot([0 1 1 0 0],[0 0 1 1 0]);disp('Mux')
  "pd{3,2}" : Outport
      mask Display: e = 0.06;c1 = [120 230 180]/255;c2 = ([120 230 180]+0)/(256+128);c3 = ([120 230 180]+128)/(256+128);patch([0 1 1 0],[0 0 1 1],c1);patch([0 1 1 1-e 1-e 0+e],[0 0 1 1-e 0+e 0+e],c2);patch([0 0 1 1-e 0+e 0+e],[0 1 1 1-e 1-e 0+e],c3);plot([0 1 1 0 0],[0 0 1 1 0]);
  wires:
  "p{3}"/1 -> mux/1
  mux/1 -> "pd{3,2}"/1

== system MCVT_Integration/Sys2 - Structural System/Sys2_Structural_System/Fault Detection and Diagnosis (FDD) with Binary Classifer/Pattern Recognition Neural Network/Segment 2 (2)/Layer 3/LW{3,2} ==
  "ad{3,2}" : Inport  PortDimensions=20
      mask Display: e = 0.06;c1 = [120 230 180]/255;c2 = ([120 230 180]+0)/(256+128);c3 = ([120 230 180]+128)/(256+128);patch([0 1 1 0],[0 0 1 1],c1);patch([0 1 1 1-e 1-e 0+e],[0 0 1 1-e 0+e 0+e],c2);patch([0 0 1 1-e 0+e 0+e],[0 1 1 1-e 1-e 0+e],c3);plot([0 1 1 0 0],[0 0 1 1 0]);
  "IW{3,2}(1,:)'" : Constant  Value=[-0.9318391516324127099579754940350539982318878173828125;-0.033545309495074775163470093275464023463428020477294921875;0.347296734017951858053407931947731412947177886962890625;0.712006626379439122587200472480617463588714599609375;0.302830260530404127283787829583161510527133941650390625;-0.71591690949835962154423896208754740655422210693359375;-0.5793954394993410250691567853209562599658966064453125;0.40260440267427355376383957263897173106670379638671875;0.8758821367775500021934931282885372638702392578125;0.08389820265766634310722338341292925179004669189453125;-0.75796568549773102319022655137814581394195556640625;-0.1646728663089549460352145615615881979465484619140625;0.1083313645565060212572205955439130775630474090576171875;-0.304578421843035462845961092170909978449344635009765625;-0.431800547237212006823625642937258817255496978759765625;0.9863165062029202179161302410648204386234283447265625;0.77683131365980051530328864828334189951419830322265625;-0.07924707732875475862943659421944175846874713897705078125;0.151576940527647752166018335628905333578586578369140625;0.8070380969475572374705052425269968807697296142578125]
      mask Display: e = 0.06;c1 = [120 150 230]/255;c2 = ([120 150 230]+0)/383;c3 = ([120 150 230]+128)/383;patch([0 1 1 0],[0 0 1 1],c1);patch([0 1 1 1-e 1-e 0+e],[0 0 1 1-e 0+e 0+e],c2);patch([0 0 1 1-e 0+e 0+e],[0 1 1 1-e 1-e 0+e],c3);plot([0 1 1 0 0],[0 0 1 1 0]);disp('weights');
  "IW{3,2}(2,:)'" : Constant  Value=[0.3103522674895966559915905236266553401947021484375;0.7837208996432381002961164995213039219379425048828125;0.108410325400192297795598506127134896814823150634765625;0.3451624979294825035225358078605495393276214599609375;0.158611805530588567680894129807711578905582427978515625;-0.6125231569615643412163308312301523983478546142578125;-0.53502774421394561077391927028656937181949615478515625;0.9494312419697779059646336463629268109798431396484375;0.670676487460840409227103009470738470554351806640625;0.276990818965088203551516699008061550557613372802734375;-0.09404997387377940498698336568850209005177021026611328125;-0.0282205309619686824096351784874059376306831836700439453125;-0.2605846140557022128092512502917088568210601806640625;0.679504272676161757971158294822089374065399169921875;0.9022888060846618873966917817597277462482452392578125;-0.5690186245396429143283967277966439723968505859375;-0.65968942184241619042239790360326878726482391357421875;0.1700453549552697618540975099676870740950107574462890625;1.03378700926403244153561900020577013492584228515625;0.4368677162499194910338928821147419512271881103515625]
      mask Display: e = 0.06;c1 = [120 150 230]/255;c2 = ([120 150 230]+0)/383;c3 = ([120 150 230]+128)/383;patch([0 1 1 0],[0 0 1 1],c1);patch([0 1 1 1-e 1-e 0+e],[0 0 1 1-e 0+e 0+e],c2);patch([0 0 1 1-e 0+e 0+e],[0 1 1 1-e 1-e 0+e],c3);plot([0 1 1 0 0],[0 0 1 1 0]);disp('weights');
  Mux : Mux  Inputs=2
      mask Display: e = 0.06;c1 = [120 150 230]/255;c2 = ([120 150 230]+0)/383;c3 = ([120 150 230]+128)/383;patch([0 1 1 0],[0 0 1 1],c1);patch([0 1 1 1-e 1-e 0+e],[0 0 1 1-e 0+e 0+e],c2);patch([0 0 1 1-e 0+e 0+e],[0 1 1 1-e 1-e 0+e],c3);plot([0 1 1 0 0],[0 0 1 1 0]);disp('Mux');
  dotprod1 : neural/Weight Functions/dotprod
  dotprod2 : neural/Weight Functions/dotprod
  "lz{3,2}" : Outport
      mask Display: e = 0.06;c1 = [120 230 180]/255;c2 = ([120 230 180]+0)/(256+128);c3 = ([120 230 180]+128)/(256+128);patch([0 1 1 0],[0 0 1 1],c1);patch([0 1 1 1-e 1-e 0+e],[0 0 1 1-e 0+e 0+e],c2);patch([0 0 1 1-e 0+e 0+e],[0 1 1 1-e 1-e 0+e],c3);plot([0 1 1 0 0],[0 0 1 1 0]);
  wires:
  "IW{3,2}(1,:)'"/1 -> dotprod1/1
  "ad{3,2}"/1 -> dotprod1/2, dotprod2/2
  dotprod1/1 -> Mux/1
  "IW{3,2}(2,:)'"/1 -> dotprod2/1
  dotprod2/1 -> Mux/2
  Mux/1 -> "lz{3,2}"/1

== system MCVT_Integration/Sys2 - Structural System/Sys2_Structural_System/Fault Detection and Diagnosis (FDD) with Binary Classifer/Pattern Recognition Neural Network/Segment 3/Layer 1/Delays 1 ==
  "p{1}" : Inport  PortDimensions=2304
      mask Display: e = 0.06;c1 = [120 230 180]/255;c2 = ([120 230 180]+0)/(256+128);c3 = ([120 230 180]+128)/(256+128);patch([0 1 1 0],[0 0 1 1],c1);patch([0 1 1 1-e 1-e 0+e],[0 0 1 1-e 0+e 0+e],c2);patch([0 0 1 1-e 0+e 0+e],[0 1 1 1-e 1-e 0+e],c3);plot([0 1 1 0 0],[0 0 1 1 0]);
  mux : Mux  Inputs=1
      mask Display: e = 0.06;c1 = [120 150 230]/255;c2 = ([120 150 230]+0)/383;c3 = ([120 150 230]+128)/383;patch([0 1 1 0],[0 0 1 1],c1);patch([0 1 1 1-e 1-e 0+e],[0 0 1 1-e 0+e 0+e],c2);patch([0 0 1 1-e 0+e 0+e],[0 1 1 1-e 1-e 0+e],c3);plot([0 1 1 0 0],[0 0 1 1 0]);disp('Mux')
  "pd{1,1}" : Outport
      mask Display: e = 0.06;c1 = [120 230 180]/255;c2 = ([120 230 180]+0)/(256+128);c3 = ([120 230 180]+128)/(256+128);patch([0 1 1 0],[0 0 1 1],c1);patch([0 1 1 1-e 1-e 0+e],[0 0 1 1-e 0+e 0+e],c2);patch([0 0 1 1-e 0+e 0+e],[0 1 1 1-e 1-e 0+e],c3);plot([0 1 1 0 0],[0 0 1 1 0]);
  wires:
  "p{1}"/1 -> mux/1
  mux/1 -> "pd{1,1}"/1
